simulink model "CDF_VSVPWM_Grid_Parallel"  (R2024b)
settings: Solver=VariableStepAuto  StartTime=0.0  StopTime=0.1  FixedStep=1e-7  MaxStep=1e-5  RelTol=1e-4
InitFcn:
  | Ts=1/10e3;
  | Udc=600;
  | C=4000e-6;
  | m=0.7;
libraries: simulink x24, spsMosfetLib x24, spsSeriesRLCBranchLib x16, spsDiodeLib x12, spsabctoAlphaBetaZeroLib x5, spsThreePhaseVIMeasurementLib x4, spsVoltageMeasurementLib x4, spsAlphaBetaZerotoabcLib x2, spsStairGeneratorLib x2, spsOnDelayLib x2, spsdq0toAlphaBetaZeroLib x2, spsBreakerLib x1
(part 4 of 5)

== matlab function CDF_VSVPWM_Grid_Parallel/VSVPWM1/生成时间调制波/Ⅴ扇区的调制信号计算/1-1/MATLAB Function ==
  data: T1 input; ta1 output; T2 input; unused input; unused1 input; unused2 input; ta2 output
function [ta1,ta2] = fcn(T1,T2,~,~,~)

ta1 =2*(T1+T2);
ta2 =0;

== matlab function CDF_VSVPWM_Grid_Parallel/VSVPWM1/生成时间调制波/Ⅴ扇区的调制信号计算/1-1/MATLAB Function1 ==
  data: T1 input; tb1 output; unused input; unused1 input; unused2 input; T5 input; tb2 output
function [tb1,tb2] = fcn(T1,~,~,~,T5)

tb1 =2*T1;
tb2 =-T5;

== matlab function CDF_VSVPWM_Grid_Parallel/VSVPWM1/大扇区判断/MATLAB Function1 ==
  data: u input; y output
function y = fcn(u)

y = u;

== matlab function CDF_VSVPWM_Grid_Parallel/VSVPWM1/生成时间调制波/Ⅴ扇区的调制信号计算/1-1/MATLAB Function2 ==
  data: unused input; tc1 output; unused1 input; unused2 input; T4 input; T5 input; tc2 output
function [tc1,tc2] = fcn(~,~,~,T4,T5)

tc2 =-(2*T4+T5);
tc1 =0;

== matlab function CDF_VSVPWM_Grid_Parallel/VSVPWM1/生成时间调制波/Ⅴ扇区的调制信号计算/1-2/MATLAB Function ==
  data: T1 input; ta1 output; T2 input; T3 input; unused input; unused1 input; ta2 output
function [ta1,ta2] = fcn(T1,T2,T3,~,~)

ta1 =2*(T1+T2+T3);
ta2 =0;

== matlab function CDF_VSVPWM_Grid_Parallel/VSVPWM1/生成时间调制波/Ⅴ扇区的调制信号计算/1-2/MATLAB Function1 ==
  data: T1 input; tb1 output; unused input; unused1 input; unused2 input; T5 input; tb2 output
function [tb1,tb2] = fcn(T1,~,~,~,T5)

tb1 =2*T1;
tb2 =-T5;

== matlab function CDF_VSVPWM_Grid_Parallel/VSVPWM1/生成时间调制波/Ⅴ扇区的调制信号计算/1-2/MATLAB Function2 ==
  data: unused input; tc1 output; unused1 input; T3 input; T4 input; T5 input; tc2 output
function [tc1,tc2] = fcn(~,~,T3,T4,T5)

tc2 =-(2*T3+2*T4+T5);
tc1 =0;

== matlab function CDF_VSVPWM_Grid_Parallel/VSVPWM1/生成时间调制波/Ⅴ扇区的调制信号计算/1-3/MATLAB Function ==
  data: T1 input; ta1 output; T2 input; T3 input; T4 input; unused input; ta2 output
function [ta1,ta2] = fcn(T1,T2,T3,T4,~)

ta1 =2*(T1+T2+T3+T4);
ta2 =0;

== matlab function CDF_VSVPWM_Grid_Parallel/VSVPWM1/生成时间调制波/Ⅴ扇区的调制信号计算/1-3/MATLAB Function1 ==
  data: T1 input; tb1 output; unused input; unused1 input; T4 input; T5 input; tb2 output
function [tb1,tb2] = fcn(T1,~,~,T4,T5)

tb1 =2*T1;
tb2 =-1*(2*T4+T5);

== matlab function CDF_VSVPWM_Grid_Parallel/VSVPWM1/大扇区判断/MATLAB Function2 ==
  data: u input; y output
function y = fcn(u)

y = u-pi/3;

== matlab function CDF_VSVPWM_Grid_Parallel/VSVPWM1/生成时间调制波/Ⅴ扇区的调制信号计算/1-3/MATLAB Function2 ==
  data: unused input; tc1 output; unused1 input; T3 input; T4 input; T5 input; tc2 output
function [tc1,tc2] = fcn(~,~,T3,T4,T5)

tc2 =-1*(2*T3+2*T4+T5);
tc1 =0;

== matlab function CDF_VSVPWM_Grid_Parallel/VSVPWM1/生成时间调制波/Ⅴ扇区的调制信号计算/1-4/MATLAB Function ==
  data: T1 input; ta1 output; T2 input; T3 input; unused input; unused1 input; ta2 output
function [ta1,ta2] = fcn(T1,T2,T3,~,~)

ta1 =2*(T1+T2+T3);
ta2 =0;

== matlab function CDF_VSVPWM_Grid_Parallel/VSVPWM1/生成时间调制波/Ⅴ扇区的调制信号计算/1-4/MATLAB Function1 ==
  data: T1 input; tb1 output; T2 input; unused input; unused1 input; T5 input; tb2 output
function [tb1,tb2] = fcn(T1,T2,~,~,T5)

tb1 =2*(T1+T2);
tb2 =-T5;

== matlab function CDF_VSVPWM_Grid_Parallel/VSVPWM1/生成时间调制波/Ⅴ扇区的调制信号计算/1-4/MATLAB Function2 ==
  data: unused input; tc1 output; T2 input; T3 input; T4 input; T5 input; tc2 output
function [tc1,tc2] = fcn(~,T2,T3,T4,T5)

tc2 =-(2*T2+2*T3+2*T4+T5);
tc1 =0;

== matlab function CDF_VSVPWM_Grid_Parallel/VSVPWM1/生成时间调制波/Ⅴ扇区的调制信号计算/1-5/MATLAB Function ==
  data: T1 input; ta1 output; T2 input; T3 input; T4 input; unused input; ta2 output
function [ta1,ta2] = fcn(T1,T2,T3,T4,~)

ta1 =2*(T1+T2+T3+T4);
ta2 =0;

== matlab function CDF_VSVPWM_Grid_Parallel/VSVPWM1/生成时间调制波/Ⅴ扇区的调制信号计算/1-5/MATLAB Function1 ==
  data: T1 input; tb1 output; T2 input; unused input; T4 input; T5 input; tb2 output
function [tb1,tb2] = fcn(T1,T2,~,T4,T5)

tb1 =2*(T1+T2);
tb2 =-(2*T4+T5);

== matlab function CDF_VSVPWM_Grid_Parallel/VSVPWM1/大扇区判断/MATLAB Function3 ==
  data: u input; y output
function y = fcn(u)

y = u-2*pi/3;

== matlab function CDF_VSVPWM_Grid_Parallel/VSVPWM1/生成时间调制波/Ⅴ扇区的调制信号计算/1-5/MATLAB Function2 ==
  data: unused input; tc1 output; T2 input; T3 input; T4 input; T5 input; tc2 output
function [tc1,tc2] = fcn(~,T2,T3,T4,T5)

tc2 =-(2*T2+2*T3+2*T4+T5);
tc1 =0;

== matlab function CDF_VSVPWM_Grid_Parallel/VSVPWM1/生成时间调制波/Ⅵ扇区的调制信号计算/2-1/MATLAB Function ==
  data: T1 input; ta1 output; unused input; unused1 input; unused2 input; T5 input; ta2 output
function [ta1,ta2] = fcn(T1,~,~,~,T5)

ta1 =2*T1;
ta2 =-T5;

== matlab function CDF_VSVPWM_Grid_Parallel/VSVPWM1/生成时间调制波/Ⅵ扇区的调制信号计算/2-1/MATLAB Function1 ==
  data: T1 input; tb1 output; T2 input; unused input; unused1 input; unused2 input; tb2 output
function [tb1,tb2] = fcn(T1,T2,~,~,~)

tb1 =2*(T1+T2);
tb2 =0;

== matlab function CDF_VSVPWM_Grid_Parallel/VSVPWM1/生成时间调制波/Ⅵ扇区的调制信号计算/2-1/MATLAB Function2 ==
  data: unused input; tc1 output; unused1 input; unused2 input; T4 input; T5 input; tc2 output
function [tc1,tc2] = fcn(~,~,~,T4,T5)

tc2 =-(2*T4+T5);
tc1 =0;

== matlab function CDF_VSVPWM_Grid_Parallel/VSVPWM1/生成时间调制波/Ⅵ扇区的调制信号计算/2-2/MATLAB Function ==
  data: T1 input; ta1 output; unused input; unused1 input; unused2 input; T5 input; ta2 output
function [ta1,ta2] = fcn(T1,~,~,~,T5)

ta1 =2*T1;
ta2 =-T5;

== matlab function CDF_VSVPWM_Grid_Parallel/VSVPWM1/生成时间调制波/Ⅵ扇区的调制信号计算/2-2/MATLAB Function1 ==
  data: T1 input; tb1 output; T2 input; T3 input; unused input; unused1 input; tb2 output
function [tb1,tb2] = fcn(T1,T2,T3,~,~)

tb1 =2*(T1+T2+T3);
tb2 =0;

== matlab function CDF_VSVPWM_Grid_Parallel/VSVPWM1/大扇区判断/MATLAB Function4 ==
  data: u input; y output
function y = fcn(u)

y = u-pi;

== matlab function CDF_VSVPWM_Grid_Parallel/VSVPWM1/生成时间调制波/Ⅵ扇区的调制信号计算/2-2/MATLAB Function2 ==
  data: unused input; tc1 output; unused1 input; T3 input; T4 input; T5 input; tc2 output
function [tc1,tc2] = fcn(~,~,T3,T4,T5)

tc2 =-(2*T3+2*T4+T5);
tc1 =0;

== matlab function CDF_VSVPWM_Grid_Parallel/VSVPWM1/生成时间调制波/Ⅵ扇区的调制信号计算/2-3/MATLAB Function ==
  data: T1 input; ta1 output; T2 input; unused input; unused1 input; T5 input; ta2 output
function [ta1,ta2] = fcn(T1,T2,~,~,T5)

ta1 =2*(T1+T2);
ta2 =-T5;

== matlab function CDF_VSVPWM_Grid_Parallel/VSVPWM1/生成时间调制波/Ⅵ扇区的调制信号计算/2-3/MATLAB Function1 ==
  data: T1 input; tb1 output; T2 input; T3 input; unused input; unused1 input; tb2 output
function [tb1,tb2] = fcn(T1,T2,T3,~,~)

tb1 =2*(T1+T2+T3);
tb2 =0;

== matlab function CDF_VSVPWM_Grid_Parallel/VSVPWM1/生成时间调制波/Ⅵ扇区的调制信号计算/2-3/MATLAB Function2 ==
  data: unused input; tc1 output; T2 input; T3 input; T4 input; T5 input; tc2 output
function [tc1,tc2] = fcn(~,T2,T3,T4,T5)

tc2 =-(2*T2+2*T3+2*T4+T5);
tc1 =0;

== matlab function CDF_VSVPWM_Grid_Parallel/VSVPWM1/生成时间调制波/Ⅵ扇区的调制信号计算/2-4/MATLAB Function ==
  data: T1 input; ta1 output; unused input; unused1 input; T4 input; T5 input; ta2 output
function [ta1,ta2] = fcn(T1,~,~,T4,T5)

ta1 =2*T1;
ta2 =-(2*T4+T5);

== matlab function CDF_VSVPWM_Grid_Parallel/VSVPWM1/大扇区判断/MATLAB Function5 ==
  data: u input; y output
function y = fcn(u)

y = u-4*pi/3;

== matlab function CDF_VSVPWM_Grid_Parallel/VSVPWM1/生成时间调制波/Ⅵ扇区的调制信号计算/2-4/MATLAB Function1 ==
  data: T1 input; tb1 output; T2 input; T3 input; T4 input; unused input; tb2 output
function [tb1,tb2] = fcn(T1,T2,T3,T4,~)

tb1 =2*(T1+T2+T3+T4);
tb2 =0;

== matlab function CDF_VSVPWM_Grid_Parallel/VSVPWM1/生成时间调制波/Ⅵ扇区的调制信号计算/2-4/MATLAB Function2 ==
  data: unused input; tc1 output; unused1 input; T3 input; T4 input; T5 input; tc2 output
function [tc1,tc2] = fcn(~,~,T3,T4,T5)

tc2 =-(2*T3+2*T4+T5);
tc1 =0;

== matlab function CDF_VSVPWM_Grid_Parallel/VSVPWM1/生成时间调制波/Ⅵ扇区的调制信号计算/2-5/MATLAB Function ==
  data: T1 input; ta1 output; T2 input; unused input; T4 input; T5 input; ta2 output
function [ta1,ta2] = fcn(T1,T2,~,T4,T5)

ta1 =2*(T1+T2);
ta2 =-(2*T4+T5);

== matlab function CDF_VSVPWM_Grid_Parallel/VSVPWM1/生成时间调制波/Ⅵ扇区的调制信号计算/2-5/MATLAB Function1 ==
  data: T1 input; tb1 output; T2 input; T3 input; T4 input; unused input; tb2 output
function [tb1,tb2] = fcn(T1,T2,T3,T4,~)

tb1 =2*(T1+T2+T3+T4);
tb2 =0;

== matlab function CDF_VSVPWM_Grid_Parallel/VSVPWM1/生成时间调制波/Ⅵ扇区的调制信号计算/2-5/MATLAB Function2 ==
  data: unused input; tc1 output; T2 input; T3 input; T4 input; T5 input; tc2 output
function [tc1,tc2] = fcn(~,T2,T3,T4,T5)

tc2 =-(2*T2+2*T3+2*T4+T5);
tc1 =0;

== matlab function CDF_VSVPWM_Grid_Parallel/VSVPWM1/矢量作用时间计算/I1/sin(pi//3+u) ==
  data: u input; y output
function y = fcn(u)

y = sin(pi/3+u);

== matlab function CDF_VSVPWM_Grid_Parallel/VSVPWM1/矢量作用时间计算/I1/sin(pi//3-u) ==
  data: u input; y output
function y = fcn(u)

y = sin(pi/3-u);

== matlab function CDF_VSVPWM_Grid_Parallel/VSVPWM1/大扇区判断/MATLAB Function6 ==
  data: u input; y output
function y = fcn(u)

y = u-5*pi/3;

== matlab function CDF_VSVPWM_Grid_Parallel/VSVPWM1/矢量作用时间计算/I2/sin(pi//3+u) ==
  data: u input; y output
function y = fcn(u)

y = sin(pi/3+u);

== matlab function CDF_VSVPWM_Grid_Parallel/VSVPWM1/矢量作用时间计算/I2/sin(pi//6+u) ==
  data: u input; y output
function y = fcn(u)

y = sin(pi/6+u);

== matlab function CDF_VSVPWM_Grid_Parallel/VSVPWM1/矢量作用时间计算/I3/sin(pi//6+u) ==
  data: u input; y output
function y = fcn(u)

y = sin(pi/6+u);

== matlab function CDF_VSVPWM_Grid_Parallel/VSVPWM1/矢量作用时间计算/I4/sin(pi//3-u) ==
  data: u input; y output
function y = fcn(u)

y = sin(pi/3-u);

== matlab function CDF_VSVPWM_Grid_Parallel/VSVPWM1/矢量作用时间计算/I4/sin(pi//6+u) ==
  data: u input; y output
function y = fcn(u)

y = sin(pi/6+u);

== matlab function CDF_VSVPWM_Grid_Parallel/VSVPWM/生成时间调制波/Ⅲ扇区的调制信号计算1/1-4/MATLAB Function ==
  data: T1 input; ta1 output; T2 input; T3 input; unused input; unused1 input; ta2 output
function [ta1,ta2] = fcn(T1,T2,T3,~,~)

ta1 =2*(T1+T2+T3);
ta2 =0;

== matlab function CDF_VSVPWM_Grid_Parallel/VSVPWM/生成时间调制波/Ⅲ扇区的调制信号计算1/1-4/MATLAB Function1 ==
  data: T1 input; tb1 output; T2 input; unused input; unused1 input; T5 input; tb2 output
function [tb1,tb2] = fcn(T1,T2,~,~,T5)

tb1 =2*(T1+T2);
tb2 =-T5;

== matlab function CDF_VSVPWM_Grid_Parallel/VSVPWM/生成时间调制波/Ⅲ扇区的调制信号计算1/1-4/MATLAB Function2 ==
  data: unused input; tc1 output; T2 input; T3 input; T4 input; T5 input; tc2 output
function [tc1,tc2] = fcn(~,T2,T3,T4,T5)

tc2 =-(2*T2+2*T3+2*T4+T5);
tc1 =0;

== matlab function CDF_VSVPWM_Grid_Parallel/VSVPWM1/小扇区判断/MATLAB Function ==
  data: u1 input; k output; u2 input
function k = fcn(u1,u2)

k = sqrt(3)*u1/u2;

== matlab function CDF_VSVPWM_Grid_Parallel/VSVPWM/生成时间调制波/Ⅲ扇区的调制信号计算1/1-5/MATLAB Function ==
  data: T1 input; ta1 output; T2 input; T3 input; T4 input; unused input; ta2 output
function [ta1,ta2] = fcn(T1,T2,T3,T4,~)

ta1 =2*(T1+T2+T3+T4);
ta2 =0;

== matlab function CDF_VSVPWM_Grid_Parallel/VSVPWM/生成时间调制波/Ⅲ扇区的调制信号计算1/1-5/MATLAB Function1 ==
  data: T1 input; tb1 output; T2 input; unused input; T4 input; T5 input; tb2 output
function [tb1,tb2] = fcn(T1,T2,~,T4,T5)

tb1 =2*(T1+T2);
tb2 =-(2*T4+T5);

== matlab function CDF_VSVPWM_Grid_Parallel/VSVPWM/生成时间调制波/Ⅲ扇区的调制信号计算1/1-5/MATLAB Function2 ==
  data: unused input; tc1 output; T2 input; T3 input; T4 input; T5 input; tc2 output
function [tc1,tc2] = fcn(~,T2,T3,T4,T5)

tc2 =-(2*T2+2*T3+2*T4+T5);
tc1 =0;

== matlab function CDF_VSVPWM_Grid_Parallel/VSVPWM/生成时间调制波/Ⅳ扇区的调制信号计算/2-1/MATLAB Function ==
  data: T1 input; ta1 output; unused input; unused1 input; unused2 input; T5 input; ta2 output
function [ta1,ta2] = fcn(T1,~,~,~,T5)

ta1 =2*T1;
ta2 =-T5;

== matlab function CDF_VSVPWM_Grid_Parallel/VSVPWM/生成时间调制波/Ⅳ扇区的调制信号计算/2-1/MATLAB Function1 ==
  data: T1 input; tb1 output; T2 input; unused input; unused1 input; unused2 input; tb2 output
function [tb1,tb2] = fcn(T1,T2,~,~,~)

tb1 =2*(T1+T2);
tb2 =0;

== matlab function CDF_VSVPWM_Grid_Parallel/VSVPWM/生成时间调制波/Ⅳ扇区的调制信号计算/2-1/MATLAB Function2 ==
  data: unused input; tc1 output; unused1 input; unused2 input; T4 input; T5 input; tc2 output
function [tc1,tc2] = fcn(~,~,~,T4,T5)

tc2 =-(2*T4+T5);
tc1 =0;

== matlab function CDF_VSVPWM_Grid_Parallel/VSVPWM/生成时间调制波/Ⅳ扇区的调制信号计算/2-2/MATLAB Function ==
  data: T1 input; ta1 output; unused input; unused1 input; unused2 input; T5 input; ta2 output
function [ta1,ta2] = fcn(T1,~,~,~,T5)

ta1 =2*T1;
ta2 =-T5;

== matlab function CDF_VSVPWM_Grid_Parallel/VSVPWM1/生成时间调制波/Ⅰ扇区的调制信号计算/1-1/MATLAB Function ==
  data: T1 input; ta1 output; T2 input; unused input; unused1 input; unused2 input; ta2 output
function [ta1,ta2] = fcn(T1,T2,~,~,~)

ta1 =2*(T1+T2);
ta2 =0;

== matlab function CDF_VSVPWM_Grid_Parallel/VSVPWM/生成时间调制波/Ⅳ扇区的调制信号计算/2-2/MATLAB Function1 ==
  data: T1 input; tb1 output; T2 input; T3 input; unused input; unused1 input; tb2 output
function [tb1,tb2] = fcn(T1,T2,T3,~,~)

tb1 =2*(T1+T2+T3);
tb2 =0;

== matlab function CDF_VSVPWM_Grid_Parallel/VSVPWM/生成时间调制波/Ⅳ扇区的调制信号计算/2-2/MATLAB Function2 ==
  data: unused input; tc1 output; unused1 input; T3 input; T4 input; T5 input; tc2 output
function [tc1,tc2] = fcn(~,~,T3,T4,T5)

tc2 =-(2*T3+2*T4+T5);
tc1 =0;

== matlab function CDF_VSVPWM_Grid_Parallel/VSVPWM/生成时间调制波/Ⅳ扇区的调制信号计算/2-3/MATLAB Function ==
  data: T1 input; ta1 output; T2 input; unused input; unused1 input; T5 input; ta2 output
function [ta1,ta2] = fcn(T1,T2,~,~,T5)

ta1 =2*(T1+T2);
ta2 =-T5;

== matlab function CDF_VSVPWM_Grid_Parallel/VSVPWM/生成时间调制波/Ⅳ扇区的调制信号计算/2-3/MATLAB Function1 ==
  data: T1 input; tb1 output; T2 input; T3 input; unused input; unused1 input; tb2 output
function [tb1,tb2] = fcn(T1,T2,T3,~,~)

tb1 =2*(T1+T2+T3);
tb2 =0;

== matlab function CDF_VSVPWM_Grid_Parallel/VSVPWM/生成时间调制波/Ⅳ扇区的调制信号计算/2-3/MATLAB Function2 ==
  data: unused input; tc1 output; T2 input; T3 input; T4 input; T5 input; tc2 output
function [tc1,tc2] = fcn(~,T2,T3,T4,T5)

tc2 =-(2*T2+2*T3+2*T4+T5);
tc1 =0;

== matlab function CDF_VSVPWM_Grid_Parallel/VSVPWM/生成时间调制波/Ⅳ扇区的调制信号计算/2-4/MATLAB Function ==
  data: T1 input; ta1 output; unused input; unused1 input; T4 input; T5 input; ta2 output
function [ta1,ta2] = fcn(T1,~,~,T4,T5)

ta1 =2*T1;
ta2 =-(2*T4+T5);

== matlab function CDF_VSVPWM_Grid_Parallel/VSVPWM/生成时间调制波/Ⅳ扇区的调制信号计算/2-4/MATLAB Function1 ==
  data: T1 input; tb1 output; T2 input; T3 input; T4 input; unused input; tb2 output
function [tb1,tb2] = fcn(T1,T2,T3,T4,~)

tb1 =2*(T1+T2+T3+T4);
tb2 =0;

== matlab function CDF_VSVPWM_Grid_Parallel/VSVPWM/生成时间调制波/Ⅳ扇区的调制信号计算/2-4/MATLAB Function2 ==
  data: unused input; tc1 output; unused1 input; T3 input; T4 input; T5 input; tc2 output
function [tc1,tc2] = fcn(~,~,T3,T4,T5)

tc2 =-(2*T3+2*T4+T5);
tc1 =0;

== matlab function CDF_VSVPWM_Grid_Parallel/VSVPWM/生成时间调制波/Ⅳ扇区的调制信号计算/2-5/MATLAB Function ==
  data: T1 input; ta1 output; T2 input; unused input; T4 input; T5 input; ta2 output
function [ta1,ta2] = fcn(T1,T2,~,T4,T5)

ta1 =2*(T1+T2);
ta2 =-(2*T4+T5);

== matlab function CDF_VSVPWM_Grid_Parallel/VSVPWM/生成时间调制波/Ⅳ扇区的调制信号计算/2-5/MATLAB Function1 ==
  data: T1 input; tb1 output; T2 input; T3 input; T4 input; unused input; tb2 output
function [tb1,tb2] = fcn(T1,T2,T3,T4,~)

tb1 =2*(T1+T2+T3+T4);
tb2 =0;

== matlab function CDF_VSVPWM_Grid_Parallel/VSVPWM1/生成时间调制波/Ⅰ扇区的调制信号计算/1-1/MATLAB Function1 ==
  data: T1 input; tb1 output; unused input; unused1 input; unused2 input; T5 input; tb2 output
function [tb1,tb2] = fcn(T1,~,~,~,T5)

tb1 =2*T1;
tb2 =-T5;

== matlab function CDF_VSVPWM_Grid_Parallel/VSVPWM/生成时间调制波/Ⅳ扇区的调制信号计算/2-5/MATLAB Function2 ==
  data: unused input; tc1 output; T2 input; T3 input; T4 input; T5 input; tc2 output
function [tc1,tc2] = fcn(~,T2,T3,T4,T5)

tc2 =-(2*T2+2*T3+2*T4+T5);
tc1 =0;

== matlab function CDF_VSVPWM_Grid_Parallel/VSVPWM/生成时间调制波/Ⅴ扇区的调制信号计算/1-1/MATLAB Function ==
  data: T1 input; ta1 output; T2 input; unused input; unused1 input; unused2 input; ta2 output
function [ta1,ta2] = fcn(T1,T2,~,~,~)

ta1 =2*(T1+T2);
ta2 =0;

== matlab function CDF_VSVPWM_Grid_Parallel/VSVPWM/生成时间调制波/Ⅴ扇区的调制信号计算/1-1/MATLAB Function1 ==
  data: T1 input; tb1 output; unused input; unused1 input; unused2 input; T5 input; tb2 output
function [tb1,tb2] = fcn(T1,~,~,~,T5)

tb1 =2*T1;
tb2 =-T5;

== matlab function CDF_VSVPWM_Grid_Parallel/VSVPWM/生成时间调制波/Ⅴ扇区的调制信号计算/1-1/MATLAB Function2 ==
  data: unused input; tc1 output; unused1 input; unused2 input; T4 input; T5 input; tc2 output
function [tc1,tc2] = fcn(~,~,~,T4,T5)

tc2 =-(2*T4+T5);
tc1 =0;

== matlab function CDF_VSVPWM_Grid_Parallel/VSVPWM/生成时间调制波/Ⅴ扇区的调制信号计算/1-2/MATLAB Function ==
  data: T1 input; ta1 output; T2 input; T3 input; unused input; unused1 input; ta2 output
function [ta1,ta2] = fcn(T1,T2,T3,~,~)

ta1 =2*(T1+T2+T3);
ta2 =0;

== matlab function CDF_VSVPWM_Grid_Parallel/VSVPWM/生成时间调制波/Ⅴ扇区的调制信号计算/1-2/MATLAB Function1 ==
  data: T1 input; tb1 output; unused input; unused1 input; unused2 input; T5 input; tb2 output
function [tb1,tb2] = fcn(T1,~,~,~,T5)

tb1 =2*T1;
tb2 =-T5;

== matlab function CDF_VSVPWM_Grid_Parallel/VSVPWM/生成时间调制波/Ⅴ扇区的调制信号计算/1-2/MATLAB Function2 ==
  data: unused input; tc1 output; unused1 input; T3 input; T4 input; T5 input; tc2 output
function [tc1,tc2] = fcn(~,~,T3,T4,T5)

tc2 =-(2*T3+2*T4+T5);
tc1 =0;

== matlab function CDF_VSVPWM_Grid_Parallel/VSVPWM/生成时间调制波/Ⅴ扇区的调制信号计算/1-3/MATLAB Function ==
  data: T1 input; ta1 output; T2 input; T3 input; T4 input; unused input; ta2 output
function [ta1,ta2] = fcn(T1,T2,T3,T4,~)

ta1 =2*(T1+T2+T3+T4);
ta2 =0;

== matlab function CDF_VSVPWM_Grid_Parallel/VSVPWM/生成时间调制波/Ⅴ扇区的调制信号计算/1-3/MATLAB Function1 ==
  data: T1 input; tb1 output; unused input; unused1 input; T4 input; T5 input; tb2 output
function [tb1,tb2] = fcn(T1,~,~,T4,T5)

tb1 =2*T1;
tb2 =-1*(2*T4+T5);

== matlab function CDF_VSVPWM_Grid_Parallel/VSVPWM/生成时间调制波/Ⅴ扇区的调制信号计算/1-3/MATLAB Function2 ==
  data: unused input; tc1 output; unused1 input; T3 input; T4 input; T5 input; tc2 output
function [tc1,tc2] = fcn(~,~,T3,T4,T5)

tc2 =-1*(2*T3+2*T4+T5);
tc1 =0;

== matlab function CDF_VSVPWM_Grid_Parallel/VSVPWM1/生成时间调制波/Ⅰ扇区的调制信号计算/1-1/MATLAB Function2 ==
  data: unused input; tc1 output; unused1 input; unused2 input; T4 input; T5 input; tc2 output
function [tc1,tc2] = fcn(~,~,~,T4,T5)

tc2 =-(2*T4+T5);
tc1 =0;

== matlab function CDF_VSVPWM_Grid_Parallel/VSVPWM/生成时间调制波/Ⅴ扇区的调制信号计算/1-4/MATLAB Function ==
  data: T1 input; ta1 output; T2 input; T3 input; unused input; unused1 input; ta2 output
function [ta1,ta2] = fcn(T1,T2,T3,~,~)

ta1 =2*(T1+T2+T3);
ta2 =0;

== matlab function CDF_VSVPWM_Grid_Parallel/VSVPWM/生成时间调制波/Ⅴ扇区的调制信号计算/1-4/MATLAB Function1 ==
  data: T1 input; tb1 output; T2 input; unused input; unused1 input; T5 input; tb2 output
function [tb1,tb2] = fcn(T1,T2,~,~,T5)

tb1 =2*(T1+T2);
tb2 =-T5;

== matlab function CDF_VSVPWM_Grid_Parallel/VSVPWM/生成时间调制波/Ⅴ扇区的调制信号计算/1-4/MATLAB Function2 ==
  data: unused input; tc1 output; T2 input; T3 input; T4 input; T5 input; tc2 output
function [tc1,tc2] = fcn(~,T2,T3,T4,T5)

tc2 =-(2*T2+2*T3+2*T4+T5);
tc1 =0;

== matlab function CDF_VSVPWM_Grid_Parallel/VSVPWM/生成时间调制波/Ⅴ扇区的调制信号计算/1-5/MATLAB Function ==
  data: T1 input; ta1 output; T2 input; T3 input; T4 input; unused input; ta2 output
function [ta1,ta2] = fcn(T1,T2,T3,T4,~)

ta1 =2*(T1+T2+T3+T4);
ta2 =0;

== matlab function CDF_VSVPWM_Grid_Parallel/VSVPWM/生成时间调制波/Ⅴ扇区的调制信号计算/1-5/MATLAB Function1 ==
  data: T1 input; tb1 output; T2 input; unused input; T4 input; T5 input; tb2 output
function [tb1,tb2] = fcn(T1,T2,~,T4,T5)

tb1 =2*(T1+T2);
tb2 =-(2*T4+T5);

== matlab function CDF_VSVPWM_Grid_Parallel/VSVPWM/生成时间调制波/Ⅴ扇区的调制信号计算/1-5/MATLAB Function2 ==
  data: unused input; tc1 output; T2 input; T3 input; T4 input; T5 input; tc2 output
function [tc1,tc2] = fcn(~,T2,T3,T4,T5)

tc2 =-(2*T2+2*T3+2*T4+T5);
tc1 =0;

== matlab function CDF_VSVPWM_Grid_Parallel/VSVPWM/生成时间调制波/Ⅵ扇区的调制信号计算/2-1/MATLAB Function ==
  data: T1 input; ta1 output; unused input; unused1 input; unused2 input; T5 input; ta2 output
function [ta1,ta2] = fcn(T1,~,~,~,T5)

ta1 =2*T1;
ta2 =-T5;

== matlab function CDF_VSVPWM_Grid_Parallel/VSVPWM/生成时间调制波/Ⅵ扇区的调制信号计算/2-1/MATLAB Function1 ==
  data: T1 input; tb1 output; T2 input; unused input; unused1 input; unused2 input; tb2 output
function [tb1,tb2] = fcn(T1,T2,~,~,~)

tb1 =2*(T1+T2);
tb2 =0;

== matlab function CDF_VSVPWM_Grid_Parallel/VSVPWM/生成时间调制波/Ⅵ扇区的调制信号计算/2-1/MATLAB Function2 ==
  data: unused input; tc1 output; unused1 input; unused2 input; T4 input; T5 input; tc2 output
function [tc1,tc2] = fcn(~,~,~,T4,T5)

tc2 =-(2*T4+T5);
tc1 =0;

== matlab function CDF_VSVPWM_Grid_Parallel/VSVPWM/生成时间调制波/Ⅵ扇区的调制信号计算/2-2/MATLAB Function ==
  data: T1 input; ta1 output; unused input; unused1 input; unused2 input; T5 input; ta2 output
function [ta1,ta2] = fcn(T1,~,~,~,T5)

ta1 =2*T1;
ta2 =-T5;

== matlab function CDF_VSVPWM_Grid_Parallel/VSVPWM1/生成时间调制波/Ⅰ扇区的调制信号计算/1-2/MATLAB Function ==
  data: T1 input; ta1 output; T2 input; T3 input; unused input; unused1 input; ta2 output
function [ta1,ta2] = fcn(T1,T2,T3,~,~)

ta1 =2*(T1+T2+T3);
ta2 =0;

== matlab function CDF_VSVPWM_Grid_Parallel/VSVPWM/生成时间调制波/Ⅵ扇区的调制信号计算/2-2/MATLAB Function1 ==
  data: T1 input; tb1 output; T2 input; T3 input; unused input; unused1 input; tb2 output
function [tb1,tb2] = fcn(T1,T2,T3,~,~)

tb1 =2*(T1+T2+T3);
tb2 =0;

== matlab function CDF_VSVPWM_Grid_Parallel/VSVPWM/生成时间调制波/Ⅵ扇区的调制信号计算/2-2/MATLAB Function2 ==
  data: unused input; tc1 output; unused1 input; T3 input; T4 input; T5 input; tc2 output
function [tc1,tc2] = fcn(~,~,T3,T4,T5)

tc2 =-(2*T3+2*T4+T5);
tc1 =0;

== matlab function CDF_VSVPWM_Grid_Parallel/VSVPWM/生成时间调制波/Ⅵ扇区的调制信号计算/2-3/MATLAB Function ==
  data: T1 input; ta1 output; T2 input; unused input; unused1 input; T5 input; ta2 output
function [ta1,ta2] = fcn(T1,T2,~,~,T5)

ta1 =2*(T1+T2);
ta2 =-T5;

== matlab function CDF_VSVPWM_Grid_Parallel/VSVPWM/生成时间调制波/Ⅵ扇区的调制信号计算/2-3/MATLAB Function1 ==
  data: T1 input; tb1 output; T2 input; T3 input; unused input; unused1 input; tb2 output
function [tb1,tb2] = fcn(T1,T2,T3,~,~)

tb1 =2*(T1+T2+T3);
tb2 =0;

== matlab function CDF_VSVPWM_Grid_Parallel/VSVPWM/生成时间调制波/Ⅵ扇区的调制信号计算/2-3/MATLAB Function2 ==
  data: unused input; tc1 output; T2 input; T3 input; T4 input; T5 input; tc2 output
function [tc1,tc2] = fcn(~,T2,T3,T4,T5)

tc2 =-(2*T2+2*T3+2*T4+T5);
tc1 =0;

== matlab function CDF_VSVPWM_Grid_Parallel/VSVPWM/生成时间调制波/Ⅵ扇区的调制信号计算/2-4/MATLAB Function ==
  data: T1 input; ta1 output; unused input; unused1 input; T4 input; T5 input; ta2 output
function [ta1,ta2] = fcn(T1,~,~,T4,T5)

ta1 =2*T1;
ta2 =-(2*T4+T5);

== matlab function CDF_VSVPWM_Grid_Parallel/VSVPWM/生成时间调制波/Ⅵ扇区的调制信号计算/2-4/MATLAB Function1 ==
  data: T1 input; tb1 output; T2 input; T3 input; T4 input; unused input; tb2 output
function [tb1,tb2] = fcn(T1,T2,T3,T4,~)

tb1 =2*(T1+T2+T3+T4);
tb2 =0;

== matlab function CDF_VSVPWM_Grid_Parallel/VSVPWM/生成时间调制波/Ⅵ扇区的调制信号计算/2-4/MATLAB Function2 ==
  data: unused input; tc1 output; unused1 input; T3 input; T4 input; T5 input; tc2 output
function [tc1,tc2] = fcn(~,~,T3,T4,T5)

tc2 =-(2*T3+2*T4+T5);
tc1 =0;

== matlab function CDF_VSVPWM_Grid_Parallel/VSVPWM/生成时间调制波/Ⅵ扇区的调制信号计算/2-5/MATLAB Function ==
  data: T1 input; ta1 output; T2 input; unused input; T4 input; T5 input; ta2 output
function [ta1,ta2] = fcn(T1,T2,~,T4,T5)

ta1 =2*(T1+T2);
ta2 =-(2*T4+T5);

== matlab function CDF_VSVPWM_Grid_Parallel/VSVPWM/生成时间调制波/Ⅵ扇区的调制信号计算/2-5/MATLAB Function1 ==
  data: T1 input; tb1 output; T2 input; T3 input; T4 input; unused input; tb2 output
function [tb1,tb2] = fcn(T1,T2,T3,T4,~)

tb1 =2*(T1+T2+T3+T4);
tb2 =0;

== matlab function CDF_VSVPWM_Grid_Parallel/VSVPWM1/生成时间调制波/Ⅰ扇区的调制信号计算/1-2/MATLAB Function1 ==
  data: T1 input; tb1 output; unused input; unused1 input; unused2 input; T5 input; tb2 output
function [tb1,tb2] = fcn(T1,~,~,~,T5)

tb1 =2*T1;
tb2 =-T5;

== matlab function CDF_VSVPWM_Grid_Parallel/VSVPWM/生成时间调制波/Ⅵ扇区的调制信号计算/2-5/MATLAB Function2 ==
  data: unused input; tc1 output; T2 input; T3 input; T4 input; T5 input; tc2 output
function [tc1,tc2] = fcn(~,T2,T3,T4,T5)

tc2 =-(2*T2+2*T3+2*T4+T5);
tc1 =0;

== matlab function CDF_VSVPWM_Grid_Parallel/VSVPWM/矢量作用时间计算/I1/sin(pi//3+u) ==
  data: u input; y output
function y = fcn(u)

y = sin(pi/3+u);

== matlab function CDF_VSVPWM_Grid_Parallel/VSVPWM/矢量作用时间计算/I1/sin(pi//3-u) ==
  data: u input; y output
function y = fcn(u)

y = sin(pi/3-u);

== matlab function CDF_VSVPWM_Grid_Parallel/VSVPWM/矢量作用时间计算/I2/sin(pi//3+u) ==
  data: u input; y output
function y = fcn(u)

y = sin(pi/3+u);

== matlab function CDF_VSVPWM_Grid_Parallel/VSVPWM/矢量作用时间计算/I2/sin(pi//6+u) ==
  data: u input; y output
function y = fcn(u)

y = sin(pi/6+u);
